simulink model "r_1f_2x_4a_r331a"  (Simulink 7.2)
settings: Solver=ode45  StartTime=0.0  StopTime=1  RelTol=1e-3
undefined symbols (not defined in the file): ROACH
libraries: xbsIndex_r4 x3197, xbsReplacements_r4 x146, xps_library x97, casper_library x89
(part 7 of 28)

== system r_1f_2x_4a_r331a/snap_rx0 ==
  data_in : Inport  OutDataType=sfix(16)  OutScaling=2^0
  oob_in : Inport  Port=2  OutDataType=sfix(16)  OutScaling=2^0
  trig : Inport  Port=3  OutDataType=sfix(16)  OutScaling=2^0
  we : Inport  Port=4  OutDataType=sfix(16)  OutScaling=2^0
  Constant : xbsIndex_r4/Constant  arith_type=Boolean  const=1  n_bits=1  bin_pt=0  explicit_period=on  period=1  equ=P=C  opselect=C  inp2=PCIN>>17  opr=+  inp1=P  carry=CIN  dbl_ovrd=off  block_type=constant
  Constant1 : xbsIndex_r4/Constant  arith_type=Boolean  const=1  n_bits=1  bin_pt=0  explicit_period=on  period=1  equ=P=C  opselect=C  inp2=PCIN>>17  opr=+  inp1=P  carry=CIN  dbl_ovrd=off  block_type=constant
  Constant13 : Constant  OutDataType=sfix(16)  OutScaling=2^0
  Constant2 : xbsIndex_r4/Constant  arith_type=Boolean  const=1  n_bits=1  bin_pt=0  explicit_period=on  period=1  equ=P=C  opselect=C  inp2=PCIN>>17  opr=+  inp1=P  carry=CIN  dbl_ovrd=off  block_type=constant
  Delay{1..3,40} (4 alike) : xbsIndex_r4/Delay  en=off  latency=2  dbl_ovrd=off  reg_retiming=on  block_type=delay
      settings: xl_area=[0, 0, 0, 0, 0, 0, 0]
  Inverter : xbsIndex_r4/Inverter  en=off  latency=0  dbl_ovrd=off  block_type=inv
      settings: xl_area=[0, 0, 0, 0, 0, 0, 0]
  Logical1 : xbsIndex_r4/Logical  logical_function=AND  inputs=2  en=off  latency=0  precision=Full  arith_type=Unsigned  n_bits=8  bin_pt=2  align_bp=on  dbl_ovrd=off  block_type=logical
      settings: xl_area=[0, 0, 0, 0, 0, 0, 0]
  Mux1 : xbsIndex_r4/Mux  inputs=2  en=off  latency=0  precision=Full  arith_type="Signed  (2's comp)"  n_bits=8  bin_pt=2  quantization=Truncate  overflow=Wrap  dbl_ovrd=off  block_type=mux
      settings: xl_area=[0, 0, 0, 0, 0, 0, 0]
  Mux2 : xbsIndex_r4/Mux  inputs=2  en=off  latency=0  precision=Full  arith_type="Signed  (2's comp)"  n_bits=8  bin_pt=2  quantization=Truncate  overflow=Wrap  dbl_ovrd=off  block_type=mux
      settings: xl_area=[0, 0, 0, 0, 0, 0, 0]
  Register : xbsIndex_r4/Register  init=0  rst=on  en=on  dbl_ovrd=off  block_type=register
      settings: xl_area=[0, 0, 0, 0, 0, 0, 0]
  Register1 : xbsIndex_r4/Register  init=0  rst=on  en=on  dbl_ovrd=off  block_type=register
      settings: xl_area=[0, 0, 0, 0, 0, 0, 0]
  Reinterpret3 : xbsIndex_r4/Reinterpret  force_arith_type=on  arith_type=Unsigned  force_bin_pt=on  bin_pt=0  block_type=reinterpret
      settings: infoedit="Changes signal type without altering the binary representation.   You can changed the signal between signed and unsigned, and relocate the binary point.<P><P>Hardware notes: In hardware this block costs nothing.<P><P>Example:  Suppose the input is 6 bits wide, signed, with 2 fractional bits, and the output is forced to unsigned with 0 fractional bits.  Then an input of -2.0 (1110.00 in binary 2's complement) becomes an output of 56 (111000 in binary)."  block_version=10.1.3
  addr : xps_library/software register  Tag=xps:sw_reg  SystemSampleTime=-1  io_dir=To Processor  arith_type=Unsigned  bitwidth=32  bin_pt=0  sample_period=1  gw_name=r_1f_2x_4a_r331a_snap_rx0_addr_user_data_in
      link override convert: arith_type=Unsigned  n_bits=32  bin_pt=0
  bram_lsb : xps_library/Shared BRAM  Tag=xps:bram  SystemSampleTime=-1  arith_type=Unsigned  addr_width=nsamples  data_width=32  data_bin_pt=0  init_vals=0  sample_rate=1
      link override b_2x_8a_r310b_snap_rx0_bram_lsb_data_out: arith_type=Unsigned
      link override convert_din: arith_type=Unsigned
      link override mem/sim_data_in: arith_type=Unsigned
      link override r_2x_8a_r310b_snap_rx0_bram_lsb_data_out: arith_type=Unsigned
      link override r_2x_8a_r311a_snap_rx0_bram_lsb_data_out: arith_type=Unsigned
      link override r_2x_8a_r311b_1_snap_rx0_bram_lsb_data_out: arith_type=Unsigned
      link override r_2x_8a_r311b_snap_rx0_bram_lsb_data_out: arith_type=Unsigned
      link override r_2x_8a_r320a_1_snap_rx0_bram_lsb_data_out: arith_type=Unsigned
      link override r_2x_8a_r320b_1_snap_rx0_bram_lsb_data_out: arith_type=Unsigned
      link override r_2x_8a_r320b_snap_rx0_bram_lsb_data_out: arith_type=Unsigned
      link override r_4f_1x_16a_r322h_snap_rx0_bram_lsb_data_out: arith_type=Unsigned
      link override r_4f_1x_16a_r322i_snap_rx0_bram_lsb_data_out: arith_type=Unsigned
      link override r_4f_1x_16a_r322j_snap_rx0_bram_lsb_data_out: arith_type=Unsigned
      link override r_4f_1x_16a_r322k_snap_rx0_bram_lsb_data_out: arith_type=Unsigned
      link override r_4f_1x_16a_r322l_snap_rx0_bram_lsb_data_out: arith_type=Unsigned
      link override r_4f_1x_16a_r322m_snap_rx0_bram_lsb_data_out: arith_type=Unsigned
      link override r_4f_2x_16a_r320b_snap_rx0_bram_lsb_data_out: arith_type=Unsigned
      link override r_4f_2x_16a_r321a_snap_rx0_bram_lsb_data_out: arith_type=Unsigned
      link override r_4f_2x_16a_r322a_snap_rx0_bram_lsb_data_out: arith_type=Unsigned
      link override r_4f_2x_16a_r322b_snap_rx0_bram_lsb_data_out: arith_type=Unsigned
      link override r_4f_2x_16a_r322c_snap_rx0_bram_lsb_data_out: arith_type=Unsigned
      link override r_4f_2x_16a_r322d_snap_rx0_bram_lsb_data_out: arith_type=Unsigned
      link override r_4f_2x_16a_r322e_snap_rx0_bram_lsb_data_out: arith_type=Unsigned
      link override r_4f_2x_16a_r322f_snap_rx0_bram_lsb_data_out: arith_type=Unsigned
      link override r_4f_2x_16a_r322g_snap_rx0_bram_lsb_data_out: arith_type=Unsigned
      link override r_4f_2x_16a_r322h_snap_rx0_bram_lsb_data_out: arith_type=Unsigned
      link override r_4f_2x_16a_r322m_snap_rx0_bram_lsb_data_out: arith_type=Unsigned
      link override r_4f_2x_16a_r323a_snap_rx0_bram_lsb_data_out: arith_type=Unsigned
      link override r_4f_2x_16a_r330a_snap_rx0_bram_lsb_data_out: arith_type=Unsigned
      link override r_4f_2x_16a_r330b_snap_rx0_bram_lsb_data_out: arith_type=Unsigned
  bram_msb : xps_library/Shared BRAM  Tag=xps:bram  SystemSampleTime=-1  arith_type=Unsigned  addr_width=nsamples  data_width=32  data_bin_pt=0  init_vals=0  sample_rate=1
      link override b_2x_8a_r310b_snap_rx0_bram_msb_data_out: arith_type=Unsigned
      link override convert_din: arith_type=Unsigned
      link override mem/sim_data_in: arith_type=Unsigned
      link override r_2x_8a_r310b_snap_rx0_bram_msb_data_out: arith_type=Unsigned
      link override r_2x_8a_r311a_snap_rx0_bram_msb_data_out: arith_type=Unsigned
      link override r_2x_8a_r311b_1_snap_rx0_bram_msb_data_out: arith_type=Unsigned
      link override r_2x_8a_r311b_snap_rx0_bram_msb_data_out: arith_type=Unsigned
      link override r_2x_8a_r320a_1_snap_rx0_bram_msb_data_out: arith_type=Unsigned
      link override r_2x_8a_r320b_1_snap_rx0_bram_msb_data_out: arith_type=Unsigned
      link override r_2x_8a_r320b_snap_rx0_bram_msb_data_out: arith_type=Unsigned
      link override r_4f_1x_16a_r322h_snap_rx0_bram_msb_data_out: arith_type=Unsigned
      link override r_4f_1x_16a_r322i_snap_rx0_bram_msb_data_out: arith_type=Unsigned
      link override r_4f_1x_16a_r322j_snap_rx0_bram_msb_data_out: arith_type=Unsigned
      link override r_4f_1x_16a_r322k_snap_rx0_bram_msb_data_out: arith_type=Unsigned
      link override r_4f_1x_16a_r322l_snap_rx0_bram_msb_data_out: arith_type=Unsigned
      link override r_4f_1x_16a_r322m_snap_rx0_bram_msb_data_out: arith_type=Unsigned
      link override r_4f_2x_16a_r320b_snap_rx0_bram_msb_data_out: arith_type=Unsigned
      link override r_4f_2x_16a_r321a_snap_rx0_bram_msb_data_out: arith_type=Unsigned
      link override r_4f_2x_16a_r322a_snap_rx0_bram_msb_data_out: arith_type=Unsigned
      link override r_4f_2x_16a_r322b_snap_rx0_bram_msb_data_out: arith_type=Unsigned
      link override r_4f_2x_16a_r322c_snap_rx0_bram_msb_data_out: arith_type=Unsigned
      link override r_4f_2x_16a_r322d_snap_rx0_bram_msb_data_out: arith_type=Unsigned
      link override r_4f_2x_16a_r322e_snap_rx0_bram_msb_data_out: arith_type=Unsigned
      link override r_4f_2x_16a_r322f_snap_rx0_bram_msb_data_out: arith_type=Unsigned
      link override r_4f_2x_16a_r322g_snap_rx0_bram_msb_data_out: arith_type=Unsigned
      link override r_4f_2x_16a_r322h_snap_rx0_bram_msb_data_out: arith_type=Unsigned
      link override r_4f_2x_16a_r322m_snap_rx0_bram_msb_data_out: arith_type=Unsigned
      link override r_4f_2x_16a_r323a_snap_rx0_bram_msb_data_out: arith_type=Unsigned
      link override r_4f_2x_16a_r330a_snap_rx0_bram_msb_data_out: arith_type=Unsigned
      link override r_4f_2x_16a_r330b_snap_rx0_bram_msb_data_out: arith_type=Unsigned
  bram_oob : xps_library/Shared BRAM  Tag=xps:bram  SystemSampleTime=-1  arith_type=Unsigned  addr_width=nsamples  data_width=32  data_bin_pt=0  init_vals=0  sample_rate=1
      link override b_2x_8a_r310b_snap_rx0_bram_oob_data_out: arith_type=Unsigned
      link override convert_din: arith_type=Unsigned
      link override mem/sim_data_in: arith_type=Unsigned
      link override r_2x_8a_r310b_snap_rx0_bram_oob_data_out: arith_type=Unsigned
      link override r_2x_8a_r311a_snap_rx0_bram_oob_data_out: arith_type=Unsigned
      link override r_2x_8a_r311b_1_snap_rx0_bram_oob_data_out: arith_type=Unsigned
      link override r_2x_8a_r311b_snap_rx0_bram_oob_data_out: arith_type=Unsigned
      link override r_2x_8a_r320a_1_snap_rx0_bram_oob_data_out: arith_type=Unsigned
      link override r_2x_8a_r320b_1_snap_rx0_bram_oob_data_out: arith_type=Unsigned
      link override r_2x_8a_r320b_snap_rx0_bram_oob_data_out: arith_type=Unsigned
      link override r_4f_1x_16a_r322h_snap_rx0_bram_oob_data_out: arith_type=Unsigned
      link override r_4f_1x_16a_r322i_snap_rx0_bram_oob_data_out: arith_type=Unsigned
      link override r_4f_1x_16a_r322j_snap_rx0_bram_oob_data_out: arith_type=Unsigned
      link override r_4f_1x_16a_r322k_snap_rx0_bram_oob_data_out: arith_type=Unsigned
      link override r_4f_1x_16a_r322l_snap_rx0_bram_oob_data_out: arith_type=Unsigned
      link override r_4f_1x_16a_r322m_snap_rx0_bram_oob_data_out: arith_type=Unsigned
      link override r_4f_2x_16a_r320b_snap_rx0_bram_oob_data_out: arith_type=Unsigned
      link override r_4f_2x_16a_r321a_snap_rx0_bram_oob_data_out: arith_type=Unsigned
      link override r_4f_2x_16a_r322a_snap_rx0_bram_oob_data_out: arith_type=Unsigned
      link override r_4f_2x_16a_r322b_snap_rx0_bram_oob_data_out: arith_type=Unsigned
      link override r_4f_2x_16a_r322c_snap_rx0_bram_oob_data_out: arith_type=Unsigned
      link override r_4f_2x_16a_r322d_snap_rx0_bram_oob_data_out: arith_type=Unsigned
      link override r_4f_2x_16a_r322e_snap_rx0_bram_oob_data_out: arith_type=Unsigned
      link override r_4f_2x_16a_r322f_snap_rx0_bram_oob_data_out: arith_type=Unsigned
      link override r_4f_2x_16a_r322g_snap_rx0_bram_oob_data_out: arith_type=Unsigned
      link override r_4f_2x_16a_r322h_snap_rx0_bram_oob_data_out: arith_type=Unsigned
      link override r_4f_2x_16a_r322m_snap_rx0_bram_oob_data_out: arith_type=Unsigned
      link override r_4f_2x_16a_r323a_snap_rx0_bram_oob_data_out: arith_type=Unsigned
      link override r_4f_2x_16a_r330a_snap_rx0_bram_oob_data_out: arith_type=Unsigned
      link override r_4f_2x_16a_r330b_snap_rx0_bram_oob_data_out: arith_type=Unsigned
  ctrl : xps_library/software register  Tag=xps:sw_reg  SystemSampleTime=-1  io_dir=From Processor  arith_type=Unsigned  bitwidth=32  bin_pt=0  sample_period=1  gw_name=r_1f_2x_4a_r331a_snap_rx0_ctrl_user_data_out
      link override b_2x_8a_r310b_snap_rx0_ctrl_user_data_out: arith_type=Unsigned  n_bits=32  bin_pt=0  period=1
      link override r_2x_8a_r310b_snap_rx0_ctrl_user_data_out: arith_type=Unsigned  n_bits=32  bin_pt=0  period=1
      link override r_2x_8a_r311a_snap_rx0_ctrl_user_data_out: arith_type=Unsigned  n_bits=32  bin_pt=0  period=1
      link override r_2x_8a_r311b_1_snap_rx0_ctrl_user_data_out: arith_type=Unsigned  n_bits=32  bin_pt=0  period=1
      link override r_2x_8a_r311b_snap_rx0_ctrl_user_data_out: arith_type=Unsigned  n_bits=32  bin_pt=0  period=1
      link override r_2x_8a_r320a_1_snap_rx0_ctrl_user_data_out: arith_type=Unsigned  n_bits=32  bin_pt=0  period=1
      link override r_2x_8a_r320b_1_snap_rx0_ctrl_user_data_out: arith_type=Unsigned  n_bits=32  bin_pt=0  period=1
      link override r_2x_8a_r320b_snap_rx0_ctrl_user_data_out: arith_type=Unsigned  n_bits=32  bin_pt=0  period=1
      link override r_4f_1x_16a_r322h_snap_rx0_ctrl_user_data_out: arith_type=Unsigned  n_bits=32  bin_pt=0  period=1
      link override r_4f_1x_16a_r322i_snap_rx0_ctrl_user_data_out: arith_type=Unsigned  n_bits=32  bin_pt=0  period=1
      link override r_4f_1x_16a_r322j_snap_rx0_ctrl_user_data_out: arith_type=Unsigned  n_bits=32  bin_pt=0  period=1
      link override r_4f_1x_16a_r322k_snap_rx0_ctrl_user_data_out: arith_type=Unsigned  n_bits=32  bin_pt=0  period=1
      link override r_4f_1x_16a_r322l_snap_rx0_ctrl_user_data_out: arith_type=Unsigned  n_bits=32  bin_pt=0  period=1
      link override r_4f_1x_16a_r322m_snap_rx0_ctrl_user_data_out: arith_type=Unsigned  n_bits=32  bin_pt=0  period=1
      link override r_4f_2x_16a_r320b_snap_rx0_ctrl_user_data_out: arith_type=Unsigned  n_bits=32  bin_pt=0  period=1
      link override r_4f_2x_16a_r321a_snap_rx0_ctrl_user_data_out: arith_type=Unsigned  n_bits=32  bin_pt=0  period=1
      link override r_4f_2x_16a_r322a_snap_rx0_ctrl_user_data_out: arith_type=Unsigned  n_bits=32  bin_pt=0  period=1
      link override r_4f_2x_16a_r322b_snap_rx0_ctrl_user_data_out: arith_type=Unsigned  n_bits=32  bin_pt=0  period=1
      link override r_4f_2x_16a_r322c_snap_rx0_ctrl_user_data_out: arith_type=Unsigned  n_bits=32  bin_pt=0  period=1
      link override r_4f_2x_16a_r322d_snap_rx0_ctrl_user_data_out: arith_type=Unsigned  n_bits=32  bin_pt=0  period=1
      link override r_4f_2x_16a_r322e_snap_rx0_ctrl_user_data_out: arith_type=Unsigned  n_bits=32  bin_pt=0  period=1
      link override r_4f_2x_16a_r322f_snap_rx0_ctrl_user_data_out: arith_type=Unsigned  n_bits=32  bin_pt=0  period=1
      link override r_4f_2x_16a_r322g_snap_rx0_ctrl_user_data_out: arith_type=Unsigned  n_bits=32  bin_pt=0  period=1
      link override r_4f_2x_16a_r322h_snap_rx0_ctrl_user_data_out: arith_type=Unsigned  n_bits=32  bin_pt=0  period=1
      link override r_4f_2x_16a_r322m_snap_rx0_ctrl_user_data_out: arith_type=Unsigned  n_bits=32  bin_pt=0  period=1
      link override r_4f_2x_16a_r323a_snap_rx0_ctrl_user_data_out: arith_type=Unsigned  n_bits=32  bin_pt=0  period=1
      link override r_4f_2x_16a_r330a_snap_rx0_ctrl_user_data_out: arith_type=Unsigned  n_bits=32  bin_pt=0  period=1
      link override r_4f_2x_16a_r330b_snap_rx0_ctrl_user_data_out: arith_type=Unsigned  n_bits=32  bin_pt=0  period=1
  en1 : xbsIndex_r4/Slice  nbits=32  boolean_output=off  mode=Lower Bit Location + Width  bit1=0  base1=LSB of Input  bit0=32  base0=LSB of Input  dbl_ovrd=off  block_type=slice
      settings: infoedit="Extracts a given range of bits from each input sample and presents it at the output.  The output type is ordinarily unsigned with binary point at zero, but can be Boolean when the slice is one bit wide.<P><P>Hardware notes: In hardware this block costs nothing."  block_version=10.1.3
  en2 : xbsIndex_r4/Slice  nbits=32  boolean_output=off  mode=Lower Bit Location + Width  bit1=0  base1=LSB of Input  bit0=0  base0=LSB of Input  dbl_ovrd=off  block_type=slice
      settings: infoedit="Extracts a given range of bits from each input sample and presents it at the output.  The output type is ordinarily unsigned with binary point at zero, but can be Boolean when the slice is one bit wide.<P><P>Hardware notes: In hardware this block costs nothing."  block_version=10.1.3
  enable : xbsIndex_r4/Slice  nbits=1  boolean_output=on  mode=Lower Bit Location + Width  bit1=0  base1=LSB of Input  bit0=0  base0=LSB of Input  dbl_ovrd=off  block_type=slice
      settings: infoedit="Extracts a given range of bits from each input sample and presents it at the output.  The output type is ordinarily unsigned with binary point at zero, but can be Boolean when the slice is one bit wide.<P><P>Hardware notes: In hardware this block costs nothing."  block_version=10.1.3
  freeze_cntr : casper_library/Misc/freeze_cntr  SystemSampleTime=-1  CounterBits=nsamples
  posedge : casper_library/Misc/posedge  Tag=Rising Edge Detector  SystemSampleTime=-1
  trig1 : xbsIndex_r4/Slice  nbits=1  boolean_output=on  mode=Lower Bit Location + Width  bit1=0  base1=LSB of Input  bit0=1  base0=LSB of Input  dbl_ovrd=off  block_type=slice
      settings: infoedit="Extracts a given range of bits from each input sample and presents it at the output.  The output type is ordinarily unsigned with binary point at zero, but can be Boolean when the slice is one bit wide.<P><P>Hardware notes: In hardware this block costs nothing."  block_version=10.1.3
  valid : xbsIndex_r4/Slice  nbits=1  boolean_output=on  mode=Lower Bit Location + Width  bit1=0  base1=LSB of Input  bit0=2  base0=LSB of Input  dbl_ovrd=off  block_type=slice
      settings: infoedit="Extracts a given range of bits from each input sample and presents it at the output.  The output type is ordinarily unsigned with binary point at zero, but can be Boolean when the slice is one bit wide.<P><P>Hardware notes: In hardware this block costs nothing."  block_version=10.1.3
  wires:
  freeze_cntr/1 -> Register1/1, Delay2/1
  Register/1 -> freeze_cntr/2
  Mux2/1 -> Logical1/1
  Inverter/1 -> Logical1/2
  Logical1/1 -> Register/2
  valid/1 -> Mux1/1
  trig1/1 -> Mux2/1
  Register1/1 -> addr/1
  Constant2/1 -> Mux1/3
  we/1 -> Mux1/2
  Mux1/1 -> freeze_cntr/1
  Constant1/1 -> Mux2/3
  Constant/1 -> Register/1
  posedge/1 -> Inverter/1, Register1/2, Register/3
  ctrl/1 -> trig1/1, valid/1, enable/1
  Constant13/1 -> ctrl/1
  freeze_cntr/2 -> Register1/3, Delay3/1
  enable/1 -> posedge/1
  trig/1 -> Mux2/2
  Reinterpret3/1 -> en1/1, en2/1
  data_in/1 -> Delay1/1
  en1/1 -> bram_msb/2
  en2/1 -> bram_lsb/2
  oob_in/1 -> Delay40/1
  Delay40/1 -> bram_oob/2
  Delay1/1 -> Reinterpret3/1
  Delay2/1 -> bram_oob/1, bram_msb/1, bram_lsb/1
  Delay3/1 -> bram_oob/3, bram_msb/3, bram_lsb/3

== system r_1f_2x_4a_r331a/snap_vacc0 ==
  din : Inport  OutDataType=sfix(16)  OutScaling=2^0
  we : Inport  Port=2  OutDataType=sfix(16)  OutScaling=2^0
  Constant2 : xbsIndex_r4/Constant  arith_type=Boolean  const=1  n_bits=1  bin_pt=0  explicit_period=on  period=1  equ=P=C  opselect=C  inp2=PCIN>>17  opr=+  inp1=P  carry=CIN  dbl_ovrd=off  block_type=constant
      settings: block_version=10.1.1
  Convert1 : xbsIndex_r4/Convert  arith_type=Unsigned  n_bits=32  bin_pt=0  quantization=Truncate  overflow=Wrap  latency=0  dbl_ovrd=off  pipeline=off  block_type=convert
      settings: block_version=10.1.3
  Delay{1..3,14} (4 alike) : xbsIndex_r4/Delay  en=off  latency=1  dbl_ovrd=off  reg_retiming=on  block_type=delay
      settings: xl_area=[0, 0, 0, 0, 0, 0, 0]
  Inverter1 : xbsIndex_r4/Inverter  en=off  latency=0  dbl_ovrd=off  block_type=inv
      settings: xl_area=[0, 0, 0, 0, 0, 0, 0]
  Mux1 : xbsIndex_r4/Mux  inputs=2  en=off  latency=0  precision=Full  arith_type="Signed  (2's comp)"  n_bits=8  bin_pt=2  quantization=Truncate  overflow=Wrap  dbl_ovrd=off  block_type=mux
      settings: xl_area=[0, 0, 0, 0, 0, 0, 0]  block_version=10.1.1
  Pulse Generator : DiscretePulseGenerator  Period=(2*2*1000)  PhaseDelay=5
  Register1 : xbsIndex_r4/Register  init=0  rst=on  en=on  dbl_ovrd=off  block_type=register
      settings: xl_area=[0, 0, 0, 0, 0, 0, 0]  block_version=10.1.1
  Reinterpret : xbsIndex_r4/Reinterpret  force_arith_type=on  arith_type=Unsigned  force_bin_pt=on  bin_pt=0  block_type=reinterpret
      settings: infoedit="Changes signal type without altering the binary representation.   You can changed the signal between signed and unsigned, and relocate the binary point.<P><P>Hardware notes: In hardware this block costs nothing.<P><P>Example:  Suppose the input is 6 bits wide, signed, with 2 fractional bits, and the output is forced to unsigned with 0 fractional bits.  Then an input of -2.0 (1110.00 in binary 2's complement) becomes an output of 56 (111000 in binary)."  block_version=10.1.1
  addr : xps_library/software register  Tag=xps:sw_reg  SystemSampleTime=-1  io_dir=To Processor  arith_type=Unsigned  bitwidth=32  bin_pt=0  sample_period=1  gw_name=r_1f_2x_4a_r331a_snap_vacc0_addr_user_data_in
      link override convert: arith_type=Unsigned  n_bits=32  bin_pt=0
  bram : xps_library/Shared BRAM  Tag=xps:bram  SystemSampleTime=-1  arith_type=Unsigned  addr_width=nsamples  data_width=32  data_bin_pt=0  init_vals=0  sample_rate=1
      link override convert_din: arith_type=Unsigned
      link override mem/sim_data_in: arith_type=Unsigned
      link override r_4f_1x_16a_r322h_snap_xeng0_bram_data_out: arith_type=Unsigned
      link override r_4f_1x_16a_r322i_snap_xeng0_bram_data_out: arith_type=Unsigned
      link override r_4f_1x_16a_r322j_snap_xeng0_bram_data_out: arith_type=Unsigned
      link override r_4f_1x_16a_r322k_snap_vacc0_bram_data_out: arith_type=Unsigned
      link override r_4f_1x_16a_r322k_snap_xeng0_bram_data_out: arith_type=Unsigned
      link override r_4f_1x_16a_r322l_snap_vacc0_bram_data_out: arith_type=Unsigned
      link override r_4f_1x_16a_r322m_snap_vacc0_bram_data_out: arith_type=Unsigned
      link override r_4f_2x_16a_r322d_snap_descramble0_bram_data_out: arith_type=Unsigned
      link override r_4f_2x_16a_r322e_snap_descramble0_bram_data_out: arith_type=Unsigned
      link override r_4f_2x_16a_r322e_snap_xeng0_bram_data_out: arith_type=Unsigned
      link override r_4f_2x_16a_r322f_snap_xeng0_bram_data_out: arith_type=Unsigned
      link override r_4f_2x_16a_r322g_snap_xeng0_bram_data_out: arith_type=Unsigned
      link override r_4f_2x_16a_r322h_snap_xeng0_bram_data_out: arith_type=Unsigned
      link override r_4f_2x_16a_r322m_snap_vacc0_bram_data_out: arith_type=Unsigned
      link override r_4f_2x_16a_r323a_snap_vacc0_bram_data_out: arith_type=Unsigned
      link override r_4f_2x_16a_r330a_snap_vacc0_bram_data_out: arith_type=Unsigned
      link override r_4f_2x_16a_r330b_snap_vacc0_bram_data_out: arith_type=Unsigned
      link override test_snap2_10_1_snap_circ_bram_lsb_data_out: arith_type=Unsigned
  ctrl : xps_library/software register  Tag=xps:sw_reg  SystemSampleTime=-1  io_dir=From Processor  arith_type=Unsigned  bitwidth=32  bin_pt=0  sample_period=1  gw_name=r_1f_2x_4a_r331a_snap_vacc0_ctrl_user_data_out
      link override r_4f_1x_16a_r322k_snap_vacc0_ctrl_user_data_out: arith_type=Unsigned  n_bits=32  bin_pt=0  period=1
      link override r_4f_1x_16a_r322l_snap_vacc0_ctrl_user_data_out: arith_type=Unsigned  n_bits=32  bin_pt=0  period=1
      link override r_4f_1x_16a_r322m_snap_vacc0_ctrl_user_data_out: arith_type=Unsigned  n_bits=32  bin_pt=0  period=1
      link override r_4f_2x_16a_r322m_snap_vacc0_ctrl_user_data_out: arith_type=Unsigned  n_bits=32  bin_pt=0  period=1
      link override r_4f_2x_16a_r323a_snap_vacc0_ctrl_user_data_out: arith_type=Unsigned  n_bits=32  bin_pt=0  period=1
      link override r_4f_2x_16a_r330a_snap_vacc0_ctrl_user_data_out: arith_type=Unsigned  n_bits=32  bin_pt=0  period=1
      link override r_4f_2x_16a_r330b_snap_vacc0_ctrl_user_data_out: arith_type=Unsigned  n_bits=32  bin_pt=0  period=1
      link override test_qdr_vacc2_snap_vacc0_ctrl_user_data_out: arith_type=Unsigned  n_bits=32  bin_pt=0  period=1
      link override test_qdr_vacc_snap_vacc0_ctrl_user_data_out: arith_type=Unsigned  n_bits=32  bin_pt=0  period=1
  enable : xbsIndex_r4/Slice  nbits=1  boolean_output=on  mode=Lower Bit Location + Width  bit1=0  base1=LSB of Input  bit0=0  base0=LSB of Input  dbl_ovrd=off  block_type=slice
      settings: infoedit="Extracts a given range of bits from each input sample and presents it at the output.  The output type is ordinarily unsigned with binary point at zero, but can be Boolean when the slice is one bit wide.<P><P>Hardware notes: In hardware this block costs nothing."  block_version=10.1.1
  freeze_cntr : Subsystem  AncestorBlock=casper_library/Misc/freeze_cntr  mask(freeze_cntr){CounterBits=nsamples}
      mask prompts: CounterBits "Counter Length (2^?)"
      mask help:
      | A freeze counter is an enabled counter which holds its final value (regardless of enables)
      | until it is reset.
      mask Help: eval('xlWeb([getenv(''MLIB_ROOT''), ''\doc\htmlblocks\sp_freeze_cntr\sp_freeze_cntr.html''])')
  posedge : casper_library/Misc/posedge  Tag=Rising Edge Detector  SystemSampleTime=-1
  valid : xbsIndex_r4/Slice  nbits=1  boolean_output=on  mode=Lower Bit Location + Width  bit1=0  base1=LSB of Input  bit0=2  base0=LSB of Input  dbl_ovrd=off  block_type=slice
      settings: infoedit="Extracts a given range of bits from each input sample and presents it at the output.  The output type is ordinarily unsigned with binary point at zero, but can be Boolean when the slice is one bit wide.<P><P>Hardware notes: In hardware this block costs nothing."  block_version=10.1.1
  fetch : Outport  OutDataType=sfix(16)  OutScaling=2^0
  wires:
  valid/1 -> Mux1/1
  Constant2/1 -> Mux1/3
  we/1 -> Mux1/2
  Mux1/1 -> freeze_cntr/1(en)
  posedge/1 -> Register1/2, freeze_cntr/2(rst)
  ctrl/1 -> enable/1, valid/1
  din/1 -> Reinterpret/1
  Reinterpret/1 -> Delay2/1
  Pulse Generator/1 -> ctrl/1
  freeze_cntr/2(we) -> Register1/3, Delay3/1
  enable/1 -> posedge/1
  freeze_cntr/1(addr) -> Register1/1, Delay1/1
  Convert1/1 -> Delay14/1
  Delay14/1 -> addr/1
  freeze_cntr/3(afull) -> Inverter1/1
  Inverter1/1 -> fetch/1
  Register1/1 -> Convert1/1
  Delay1/1 -> bram/1
  Delay2/1 -> bram/2
  Delay3/1 -> bram/3

== system r_1f_2x_4a_r331a/snap_vacc1 ==
  din : Inport  OutDataType=sfix(16)  OutScaling=2^0
  we : Inport  Port=2  OutDataType=sfix(16)  OutScaling=2^0
  Constant2 : xbsIndex_r4/Constant  arith_type=Boolean  const=1  n_bits=1  bin_pt=0  explicit_period=on  period=1  equ=P=C  opselect=C  inp2=PCIN>>17  opr=+  inp1=P  carry=CIN  dbl_ovrd=off  block_type=constant
      settings: block_version=10.1.1
  Convert1 : xbsIndex_r4/Convert  arith_type=Unsigned  n_bits=32  bin_pt=0  quantization=Truncate  overflow=Wrap  latency=0  dbl_ovrd=off  pipeline=off  block_type=convert
      settings: block_version=10.1.3
  Delay{1..3,14} (4 alike) : xbsIndex_r4/Delay  en=off  latency=1  dbl_ovrd=off  reg_retiming=on  block_type=delay
      settings: xl_area=[0, 0, 0, 0, 0, 0, 0]
  Inverter1 : xbsIndex_r4/Inverter  en=off  latency=0  dbl_ovrd=off  block_type=inv
      settings: xl_area=[0, 0, 0, 0, 0, 0, 0]
  Mux1 : xbsIndex_r4/Mux  inputs=2  en=off  latency=0  precision=Full  arith_type="Signed  (2's comp)"  n_bits=8  bin_pt=2  quantization=Truncate  overflow=Wrap  dbl_ovrd=off  block_type=mux
      settings: xl_area=[0, 0, 0, 0, 0, 0, 0]  block_version=10.1.1
  Pulse Generator : DiscretePulseGenerator  Period=(2*2*1000)  PhaseDelay=5
  Register1 : xbsIndex_r4/Register  init=0  rst=on  en=on  dbl_ovrd=off  block_type=register
      settings: xl_area=[0, 0, 0, 0, 0, 0, 0]  block_version=10.1.1
  Reinterpret : xbsIndex_r4/Reinterpret  force_arith_type=on  arith_type=Unsigned  force_bin_pt=on  bin_pt=0  block_type=reinterpret
      settings: infoedit="Changes signal type without altering the binary representation.   You can changed the signal between signed and unsigned, and relocate the binary point.<P><P>Hardware notes: In hardware this block costs nothing.<P><P>Example:  Suppose the input is 6 bits wide, signed, with 2 fractional bits, and the output is forced to unsigned with 0 fractional bits.  Then an input of -2.0 (1110.00 in binary 2's complement) becomes an output of 56 (111000 in binary)."  block_version=10.1.1
  addr : xps_library/software register  Tag=xps:sw_reg  SystemSampleTime=-1  io_dir=To Processor  arith_type=Unsigned  bitwidth=32  bin_pt=0  sample_period=1  gw_name=r_1f_2x_4a_r331a_snap_vacc1_addr_user_data_in
      link override convert: arith_type=Unsigned  n_bits=32  bin_pt=0
  bram : xps_library/Shared BRAM  Tag=xps:bram  SystemSampleTime=-1  arith_type=Unsigned  addr_width=nsamples  data_width=32  data_bin_pt=0  init_vals=0  sample_rate=1
      link override convert_din: arith_type=Unsigned
      link override mem/sim_data_in: arith_type=Unsigned
      link override r_4f_1x_16a_r322h_snap_xeng0_bram_data_out: arith_type=Unsigned
      link override r_4f_1x_16a_r322i_snap_xeng0_bram_data_out: arith_type=Unsigned
      link override r_4f_1x_16a_r322j_snap_xeng0_bram_data_out: arith_type=Unsigned
      link override r_4f_1x_16a_r322k_snap_vacc0_bram_data_out: arith_type=Unsigned
      link override r_4f_1x_16a_r322k_snap_xeng0_bram_data_out: arith_type=Unsigned
      link override r_4f_1x_16a_r322l_snap_vacc0_bram_data_out: arith_type=Unsigned
      link override r_4f_1x_16a_r322m_snap_vacc0_bram_data_out: arith_type=Unsigned
      link override r_4f_2x_16a_r322d_snap_descramble0_bram_data_out: arith_type=Unsigned
      link override r_4f_2x_16a_r322e_snap_descramble0_bram_data_out: arith_type=Unsigned
      link override r_4f_2x_16a_r322e_snap_xeng0_bram_data_out: arith_type=Unsigned
      link override r_4f_2x_16a_r322f_snap_xeng0_bram_data_out: arith_type=Unsigned
      link override r_4f_2x_16a_r322g_snap_xeng0_bram_data_out: arith_type=Unsigned
      link override r_4f_2x_16a_r322h_snap_xeng0_bram_data_out: arith_type=Unsigned
      link override r_4f_2x_16a_r322m_snap_vacc0_bram_data_out: arith_type=Unsigned
      link override r_4f_2x_16a_r322m_snap_vacc1_bram_data_out: arith_type=Unsigned
      link override r_4f_2x_16a_r323a_snap_vacc1_bram_data_out: arith_type=Unsigned
      link override r_4f_2x_16a_r330a_snap_vacc1_bram_data_out: arith_type=Unsigned
      link override r_4f_2x_16a_r330b_snap_vacc1_bram_data_out: arith_type=Unsigned
      link override test_snap2_10_1_snap_circ_bram_lsb_data_out: arith_type=Unsigned
  ctrl : xps_library/software register  Tag=xps:sw_reg  SystemSampleTime=-1  io_dir=From Processor  arith_type=Unsigned  bitwidth=32  bin_pt=0  sample_period=1  gw_name=r_1f_2x_4a_r331a_snap_vacc1_ctrl_user_data_out
      link override r_4f_1x_16a_r322k_snap_vacc0_ctrl_user_data_out: arith_type=Unsigned  n_bits=32  bin_pt=0  period=1
      link override r_4f_1x_16a_r322l_snap_vacc0_ctrl_user_data_out: arith_type=Unsigned  n_bits=32  bin_pt=0  period=1
      link override r_4f_1x_16a_r322m_snap_vacc0_ctrl_user_data_out: arith_type=Unsigned  n_bits=32  bin_pt=0  period=1
      link override r_4f_2x_16a_r322m_snap_vacc0_ctrl_user_data_out: arith_type=Unsigned  n_bits=32  bin_pt=0  period=1
      link override r_4f_2x_16a_r322m_snap_vacc1_ctrl_user_data_out: arith_type=Unsigned  n_bits=32  bin_pt=0  period=1
      link override r_4f_2x_16a_r323a_snap_vacc1_ctrl_user_data_out: arith_type=Unsigned  n_bits=32  bin_pt=0  period=1
      link override r_4f_2x_16a_r330a_snap_vacc1_ctrl_user_data_out: arith_type=Unsigned  n_bits=32  bin_pt=0  period=1
      link override r_4f_2x_16a_r330b_snap_vacc1_ctrl_user_data_out: arith_type=Unsigned  n_bits=32  bin_pt=0  period=1
      link override test_qdr_vacc2_snap_vacc0_ctrl_user_data_out: arith_type=Unsigned  n_bits=32  bin_pt=0  period=1
      link override test_qdr_vacc_snap_vacc0_ctrl_user_data_out: arith_type=Unsigned  n_bits=32  bin_pt=0  period=1
  enable : xbsIndex_r4/Slice  nbits=1  boolean_output=on  mode=Lower Bit Location + Width  bit1=0  base1=LSB of Input  bit0=0  base0=LSB of Input  dbl_ovrd=off  block_type=slice
      settings: infoedit="Extracts a given range of bits from each input sample and presents it at the output.  The output type is ordinarily unsigned with binary point at zero, but can be Boolean when the slice is one bit wide.<P><P>Hardware notes: In hardware this block costs nothing."  block_version=10.1.1
  freeze_cntr : Subsystem  AncestorBlock=casper_library/Misc/freeze_cntr  mask(freeze_cntr){CounterBits=nsamples}
      mask prompts: CounterBits "Counter Length (2^?)"
      mask help:
      | A freeze counter is an enabled counter which holds its final value (regardless of enables)
      | until it is reset.
      mask Help: eval('xlWeb([getenv(''MLIB_ROOT''), ''\doc\htmlblocks\sp_freeze_cntr\sp_freeze_cntr.html''])')
  posedge : casper_library/Misc/posedge  Tag=Rising Edge Detector  SystemSampleTime=-1
  valid : xbsIndex_r4/Slice  nbits=1  boolean_output=on  mode=Lower Bit Location + Width  bit1=0  base1=LSB of Input  bit0=2  base0=LSB of Input  dbl_ovrd=off  block_type=slice
      settings: infoedit="Extracts a given range of bits from each input sample and presents it at the output.  The output type is ordinarily unsigned with binary point at zero, but can be Boolean when the slice is one bit wide.<P><P>Hardware notes: In hardware this block costs nothing."  block_version=10.1.1
  fetch : Outport  OutDataType=sfix(16)  OutScaling=2^0
  wires:
  Delay3/1 -> bram/3
  Delay2/1 -> bram/2
  Delay1/1 -> bram/1
  Register1/1 -> Convert1/1
  Inverter1/1 -> fetch/1
  freeze_cntr/3(afull) -> Inverter1/1
  Delay14/1 -> addr/1
  Convert1/1 -> Delay14/1
  freeze_cntr/1(addr) -> Delay1/1, Register1/1
  enable/1 -> posedge/1
  freeze_cntr/2(we) -> Delay3/1, Register1/3
  Pulse Generator/1 -> ctrl/1
  Reinterpret/1 -> Delay2/1
  din/1 -> Reinterpret/1
  ctrl/1 -> valid/1, enable/1
  posedge/1 -> freeze_cntr/2(rst), Register1/2
  Mux1/1 -> freeze_cntr/1(en)
  we/1 -> Mux1/2
  Constant2/1 -> Mux1/3
  valid/1 -> Mux1/1
